simulink model "ofdm_txrx_supermimo"  (Simulink 7.1)
settings: Solver=VariableStepDiscrete  SolverMode=SingleTasking  StartTime=0.0  StopTime=simOnly_simLength  RelTol=1e-3
PostLoadFcn:
  | ofdm_rx_supermimo_init
InitFcn:
  | ofdm_rx_supermimo_init
StartFcn:
  | ofdm_rx_supermimo_init
values from neighbouring files (symbols this model uses):
  Action0_Config = 0* 31*2^18 + 0*2^24 + PHY_AUTORESPONSE_REQ_GOODHDR + PHY_AUTORESPONSE_REQ_GOODPKT + PHY_AUTORESPONSE_REQ_FLAGA    [ofdm_tx_supermimo_init.m]
  Action1_Config = 0* 62*2^18 + PHY_AUTORESPONSE_REQ_GOODHDR + PHY_AUTORESPONSE_REQ_GOODPKT    [ofdm_tx_supermimo_init.m]
  Action2_Config = 0    [ofdm_tx_supermimo_init.m]
  Action3_Config = 0    [ofdm_tx_supermimo_init.m]
  Action4_Config = 0    [ofdm_tx_supermimo_init.m]
  Action5_Config = 0    [ofdm_tx_supermimo_init.m]
  ActionID_AFTransmit = 31    [ofdm_tx_supermimo_init.m]
  ActionID_Disabled = 0    [ofdm_tx_supermimo_init.m]
  ActionID_SetFlagA = 62    [ofdm_tx_supermimo_init.m]
  ActionID_SetFlagB = 61    [ofdm_tx_supermimo_init.m]
  BER_RAM_init_size = 2048    [ofdm_tx_supermimo_init.m]
  CPLength = 16    [ofdm_tx_supermimo_init.m]
  longCorr_coef_i = [-4*real(LTS) 0]    [ofdm_rx_supermimo_init.m]
  longCorr_coef_q = [4*imag(LTS) 0]    [ofdm_rx_supermimo_init.m]
  Match0_Config = 0    [ofdm_tx_supermimo_init.m]
  Match1_Config = 0    [ofdm_tx_supermimo_init.m]
  Match2_Config = 0    [ofdm_tx_supermimo_init.m]
  Match3_Config = 0    [ofdm_tx_supermimo_init.m]
  Match4_Config = 0    [ofdm_tx_supermimo_init.m]
  Match5_Config = 0    [ofdm_tx_supermimo_init.m]
  Match6_Config = 0    [ofdm_tx_supermimo_init.m]
  Match7_Config = 0    [ofdm_tx_supermimo_init.m]
  max_CP_length = 16    [ofdm_tx_supermimo_init.m]
  max_num_baseRateSymbols = 31    [ofdm_tx_supermimo_init.m]
  max_num_subcarriers = 64    [ofdm_tx_supermimo_init.m]
  max_num_trainingSymbols = 15    [ofdm_tx_supermimo_init.m]
  max_numBytes = 16384    [ofdm_tx_supermimo_init.m]
  max_OFDM_symbols = 511    [ofdm_tx_supermimo_init.m]
  modConstellation_qam16 = .75*[1 3 -1 -3]./3    [ofdm_tx_supermimo_init.m]
  modConstellation_qam64 = 0.875*[3 1 5 7 -3 -1 -5 -7]./7    [ofdm_tx_supermimo_init.m]
  modConstellation_qpsk = [1 -1]./sqrt(2)    [ofdm_tx_supermimo_init.m]
  numBaseRateSymbols = ceil(numHeaderBytes / numBytes_BaseRateOFDMSymbol)    [ofdm_tx_supermimo_init.m]
  numSubcarriers = 64    [ofdm_tx_supermimo_init.m]
  numTrainingSymbols = 2    [ofdm_tx_supermimo_init.m]
  pilot_indicies = 7 + ( (64-21) * 2^8) + ( (64-7) * 2^16) + (21 * 2^24)    (= 356068103)    [ofdm_tx_supermimo_init.m]
  pilotValues = hex2dec('A57D') + (2^16 * hex2dec('5A82'))    [ofdm_tx_supermimo_init.m]
  pkt_crossCorr_thresh = 0.7    [ofdm_rx_supermimo_init.m]
  pkt_energy_thresh = 0    [ofdm_rx_supermimo_init.m]
  pktByteNums = numHeaderBytes +  				byteIndex_numPayloadBytes(1)*2^8 +  				byteIndex_numPayloadBytes(2)*2^16 +  				byteIndex_simpleDynModMasks * 2^24    [ofdm_rx_supermimo_init.m]
  PktDet_Delay = 58+32 +      Symbol_Timing_Offset*2^7 +      0*2^16 +      (117* 2^24)    (= 1962935642)    [ofdm_rx_supermimo_init.m]
  pktTiming_count_payloadStart = pktTiming_count_lsStart + pktTiming_ls_num*pktTiming_ls_length    [ofdm_rx_supermimo_init.m]
  preamble = preamble_scale*[shortsyms_10 longsyms_2]    [ofdm_tx_supermimo_init.m]
  preamble_ROM = preamble_I_ROM + preamble_Q_ROM*2^16    [ofdm_tx_supermimo_init.m]
  RAM_init_size = max_numBytes    (= 16384)    [ofdm_tx_supermimo_init.m]
  reg_AF_Tx_Blanking = (320+80) + ( (320+80+79)*2^16)    (= 31392144)    [ofdm_rx_supermimo_init.m]
  reg_AF_Tx_Scaling = 2944    [ofdm_rx_supermimo_init.m]
  reg_Interrupt_PktBuf_Control = reg_InterruptControl +  	1 * 2^16 +  	0 * 2^24    (= 65656)    [ofdm_rx_supermimo_init.m]
  reg_PilotCalcParams = (4 * 2^16) + (minPilotChanMag)    (= 262184.96)    [ofdm_rx_supermimo_init.m]
  regRx_chanEst_minMag = ((0.5)*2^15) + ((0.5 * 2^15)*2^16)    (= 1073758208)    [ofdm_rx_supermimo_init.m]
  regRx_coarseCalcCorrection = round((1.3e-3 - 1.2982e-3)*2^32)    [ofdm_rx_supermimo_init.m]
  regRx_pilotCalcCorrection = round(1.00115 * 2^31)    [ofdm_rx_supermimo_init.m]
  regRx_preCFOoptions = 0 * 2^0 +  	1 * 2^1 +  	0    (= 2)    [ofdm_rx_supermimo_init.m]
  rx_controlBits = 1 * 2  		 + 1 * 4  		 + 0 * 8  		 + 1 * 16 * rx_SISO_Mode  		 + 1 * 32  		 + 1 * 64  		 + 1 * 128  		 + 1 * 256  		 + 1 * 512  		 + 1 * 1024  		 + 1 * 4096  		 + 0 * 16384  		 + 0 * 32768  		 + 1 * 65536  		 + 0 * 131072  		 + 0 * 262144  		 + 1 * 524288  		 + 1 * 1048576 * tx_Alamouti_Mode  		 + 0 * 2097152           + 1 * 33554432  		 + 0 * 4194304  		 + 0 * 8388608  		 + 0 * 16777216  		 + 1 *  ...(+49 chars)    [ofdm_rx_supermimo_init.m]
  Rx_PktDet_LongCorr_Params = LongCorrCounterLoad + 0*2^8 + 0*2^16 + 255*2^24    (= 4278190325)    [ofdm_rx_supermimo_init.m]
  Rx_PktDet_LongCorrThresholds = 8000    [ofdm_rx_supermimo_init.m]
  rx_postEq_scaling = round(rxScaling*2^11) * (1 + 2^16)    [ofdm_rx_supermimo_init.m]
  RxDataScrambling_Seq = [40 198 78 63 82 173 102 245 48 111 172 115 147 230 216 93 72 65 62 2 205 242 122 90 128 83 105 97 73 10 5 252]    [ofdm_tx_supermimo_init.m]
  RxReg_FixedPktLen = 0    [ofdm_rx_supermimo_init.m]
  shortSymbol_time = shortSymbol_time(1:16).'    [ofdm_tx_supermimo_init.m]
  simOnly_numSamples = length(preamble)+( (numSubcarriers+CPLength)*(numTrainingSymbols + numBaseRateSymbols + numFullRateSymbols/2) )    [ofdm_tx_supermimo_init.m]
  train = [train train]    [ofdm_tx_supermimo_init.m]
  tx_controlBits = (antB_preambleShift * 2^4) + (2^1 * tx_Alamouti_Mode) +  	(2^0 * tx_SISO_Mode) + 4*0 + 8*1 + 0*256 + 512*0 + 1024*0 +  	0*hex2dec('1000') + 0*hex2dec('2000') + 0*hex2dec('4000') +  	1*hex2dec('8000') + 1*hex2dec('10000') + hex2dec('20000') +  	16*hex2dec('100000')    [ofdm_tx_supermimo_init.m]
  tx_delays = (50*2^0) + (0*2^8) + (30*2^12)    (= 122930)    [ofdm_tx_supermimo_init.m]
  tx_scaling = (3072) + (12288 * 2^16)    (= 805309440)    [ofdm_tx_supermimo_init.m]
  TxDataScrambling_Seq = [40 198 78 63 82 173 102 245 48 111 172 115 147 230 216 93 72 65 62 2 205 242 122 90 128 83 105 97 73 10 5 252]    [ofdm_tx_supermimo_init.m]
  txfilt_firpm = 0.95*txfilt_firpm./(max(txfilt_firpm))    [ofdm_tx_supermimo_init.m]
  txfilt_intfilt = [0 intfilt(4,4,.8)]    [ofdm_tx_supermimo_init.m]
  txReg_symbolCounts = (2^8 *numBaseRateSymbols ) + numTrainingSymbols    [ofdm_tx_supermimo_init.m]
  TxRx_FFTScaling = (3 * 2^0) +  	(2 * 2^2) +  	(1 * 2^4) +  	(1 * 2^6) +  	(1 * 2^8) +  	(0 * 2^10)    (= 347)    [ofdm_tx_supermimo_init.m]
undefined symbols (not defined in the file or in its folder): AntA, AntB, BaseRate, Down, gcbh, numBuffs, numBytes, Up, xlBlockLoadCallback, xlGetSimulinkPeriod, xlipgetpartsetting
libraries: xbsIndex_r4 x6035, simulink x4, dspsigops x4, commchan3 x2, dspsrcs4 x2, commrflib2 x2
(part 20 of 61)

== system ofdm_txrx_supermimo/OFDM Rx MIMO/FFT & Chan Est/Addr Gen1/Oscillator ==
  en : Inport  OutDataType=sfix(16)  OutScaling=2^0
  rst : Inport  Port=2  OutDataType=sfix(16)  OutScaling=2^0
  Assert : xbsIndex_r4/Assert  Orientation=left  assert_type=on  type_source=Explicitly  arith_type=Boolean  n_bits=16  bin_pt=14  assert_rate=on  rate_source=Explicitly  period=2  output_port=on  block_type=assert
      settings: infoedit=Asserts a user-defined sample rate and/or type on a signal.<P><P>Hardware notes: In hardware this block costs nothing.  block_version=VER_STRING_GOES_HERE
  Inverter1 : xbsIndex_r4/Inverter  Orientation=left  en=off  latency=0  dbl_ovrd=off  block_type=inv
      settings: block_version=VER_STRING_GOES_HERE
  Register : xbsIndex_r4/Register  init=0  rst=on  en=on  dbl_ovrd=off  block_type=register
      settings: block_version=VER_STRING_GOES_HERE
  Q : Outport  OutDataType=sfix(16)  OutScaling=2^0
  wires:
  Register/1 -> Q/1, Assert/1
  en/1 -> Register/3
  Inverter1/1 -> Register/1
  rst/1 -> Register/2
  Assert/1 -> Inverter1/1

== system ofdm_txrx_supermimo/OFDM Rx MIMO/FFT & Chan Est/Addr Gen1/Output Sym Counter ==
  FFT Done : Inport  OutDataType=sfix(16)  OutScaling=2^0
  Not Training : Inport  Port=2  OutDataType=sfix(16)  OutScaling=2^0
  Payload : Inport  Port=3  OutDataType=sfix(16)  OutScaling=2^0
  Read Addr : Inport  Port=4  OutDataType=sfix(16)  OutScaling=2^0
  Constant1 : xbsIndex_r4/Constant  arith_type=Unsigned  const=numSubcarriers-1  n_bits=ceil(log2(numSubcarriers))  bin_pt=0  explicit_period=off  period=1  equ=P=C  opselect=C  inp2=PCIN>>17  opr=+  inp1=P  carry=CIN  dbl_ovrd=off  block_type=constant
      settings: block_version=VER_STRING_GOES_HERE
  Counter1 : xbsIndex_r4/Counter  cnt_type=Free Running  cnt_to=127  operation=Up  start_count=0  cnt_by_val=1  arith_type=Unsigned  n_bits=ceil(log2(max_OFDM_symbols))  bin_pt=0  load_pin=off  rst=on  en=on  explicit_period=off  period=2  dbl_ovrd=off  use_behavioral_HDL=off  use_rpm=on  implementation=Fabric  block_type=counter
      settings: xl_area=[6 12 0 12 0 0 0]  block_version=VER_STRING_GOES_HERE
  Inverter3 : xbsIndex_r4/Inverter  en=off  latency=0  dbl_ovrd=off  block_type=inv
      settings: block_version=VER_STRING_GOES_HERE
  Logical1 : xbsIndex_r4/Logical  logical_function=AND  inputs=2  en=off  latency=0  precision=Full  arith_type=Unsigned  n_bits=8  bin_pt=2  align_bp=on  dbl_ovrd=off  block_type=logical
      settings: xl_area=[0, 0, 0, 0, 0, 0, 0]  block_version=VER_STRING_GOES_HERE
  Logical2 : xbsIndex_r4/Logical  logical_function=OR  inputs=2  en=off  latency=0  precision=Full  arith_type=Unsigned  n_bits=8  bin_pt=2  align_bp=on  dbl_ovrd=off  block_type=logical
      settings: xl_area=[0, 0, 0, 0, 0, 0, 0]  block_version=VER_STRING_GOES_HERE
  Logical3 : xbsIndex_r4/Logical  logical_function=AND  inputs=2  en=off  latency=0  precision=Full  arith_type=Unsigned  n_bits=8  bin_pt=2  align_bp=on  dbl_ovrd=off  block_type=logical
      settings: xl_area=[0, 0, 0, 0, 0, 0, 0]  block_version=VER_STRING_GOES_HERE
  Logical4 : xbsIndex_r4/Logical  logical_function=AND  inputs=2  en=off  latency=0  precision=Full  arith_type=Unsigned  n_bits=8  bin_pt=2  align_bp=on  dbl_ovrd=off  block_type=logical
      settings: xl_area=[0, 0, 0, 0, 0, 0, 0]  block_version=VER_STRING_GOES_HERE
  Posedge : Subsystem
  Posedge1 : Subsystem
  Posedge2 : Subsystem
  Posedge3 : Subsystem
  Relational : xbsIndex_r4/Relational  mode=a=b  en=off  latency=0  dbl_ovrd=off  block_type=relational
      settings: block_version=VER_STRING_GOES_HERE
  S-R Latch2 : Subsystem
  Sym Index : Outport  OutDataType=sfix(16)  OutScaling=2^0
  wires:
  Logical4/1 -> Logical3/2
  S-R Latch2/1(D) -> Logical4/2
  Posedge/1(Q) -> S-R Latch2/1(S), Logical4/1
  FFT Done/1 -> Posedge/1(D)
  Counter1/1 -> Sym Index/1
  Not Training/1 -> Inverter3/1, Logical1/2
  Inverter3/1 -> Logical3/1
  Payload/1 -> Posedge1/1(D)
  Posedge1/1(Q) -> S-R Latch2/2(R), Counter1/1
  Read Addr/1 -> Relational/2
  Constant1/1 -> Relational/1
  Relational/1 -> Logical1/1
  Logical1/1 -> Posedge2/1(D)
  Logical3/1 -> Posedge3/1(D)
  Logical2/1 -> Counter1/2
  Posedge2/1(Q) -> Logical2/1
  Posedge3/1(Q) -> Logical2/2

== system ofdm_txrx_supermimo/OFDM Rx MIMO/FFT & Chan Est/Addr Gen1/Rd Delay ==
  note: Delay the equalizer inputs in Alamout mode in order
      | to calculate pilot phaes for both r0 and r1 before
      | starting the decoding process. In SISO/Multiplexing mode,
      | each symbol has its own pilots, so there's a shorter delay.
  D : Inport
  Delay1 : xbsIndex_r4/Delay  en=off  latency=3  dbl_ovrd=off  reg_retiming=off  block_type=delay
      settings: block_version=10.1.2
  Delay2 : xbsIndex_r4/Delay  en=off  latency=35  dbl_ovrd=off  reg_retiming=off  block_type=delay
      settings: block_version=10.1.2
  Inverter1 : xbsIndex_r4/Inverter  en=off  latency=0  dbl_ovrd=off  block_type=inv
  Logical1 : xbsIndex_r4/Logical  logical_function=AND  inputs=2  en=off  latency=0  precision=Full  arith_type=Unsigned  n_bits=16  bin_pt=0  align_bp=on  dbl_ovrd=off  block_type=logical
  Logical2 : xbsIndex_r4/Logical  logical_function=OR  inputs=2  en=off  latency=0  precision=Full  arith_type=Unsigned  n_bits=16  bin_pt=0  align_bp=on  dbl_ovrd=off  block_type=logical
  Logical3 : xbsIndex_r4/Logical  logical_function=AND  inputs=2  en=off  latency=0  precision=Full  arith_type=Unsigned  n_bits=16  bin_pt=0  align_bp=on  dbl_ovrd=off  block_type=logical
  Q : Outport
  wires:
  Logical1/1 -> Logical2/1
  Logical3/1 -> Logical2/2
  Logical2/1 -> Q/1
  [regRx_AlamoutiMode] -> Logical1/2, Inverter1/1
  Inverter1/1 -> Logical3/1
  Delay2/1 -> Logical1/1
  D/1 -> Delay2/1, Delay1/1
  Delay1/1 -> Logical3/2

== system ofdm_txrx_supermimo/OFDM Rx MIMO/FFT & Chan Est/Addr Gen1/S-R Latch1 ==
  S : Inport  OutDataType=sfix(16)  OutScaling=2^0
  R : Inport  Port=2  OutDataType=sfix(16)  OutScaling=2^0
  Constant2 : xbsIndex_r4/Constant  arith_type=Boolean  const=1  n_bits=1  bin_pt=0  explicit_period=off  period=1  equ=P=C  opselect=C  inp2=PCIN>>17  opr=+  inp1=P  carry=CIN  dbl_ovrd=off  block_type=constant
      settings: block_version=VER_STRING_GOES_HERE
  Register : xbsIndex_r4/Register  init=0  rst=on  en=on  dbl_ovrd=off  block_type=register
      settings: xl_area=[0, 0, 0, 0, 0, 0, 0]  block_version=VER_STRING_GOES_HERE
  D : Outport  OutDataType=sfix(16)  OutScaling=2^0
  wires:
  Register/1 -> D/1
  S/1 -> Register/3
  R/1 -> Register/2
  Constant2/1 -> Register/1

== system ofdm_txrx_supermimo/OFDM Rx MIMO/FFT & Chan Est/Addr_Generator/Initial Read Addr Timing Calc ==
  note: The FFT runs 4x as fast as the input samples. As the input
      | samples are written to the buffers, the FFT waits to start
      | until it can have access to the newest sample it needs
      | 1 cycle after it's written.
  AddSub1 : xbsIndex_r4/AddSub  mode=Addition  use_carryin=off  use_carryout=off  en=off  latency=0  precision=User Defined  arith_type=Unsigned  n_bits=ceil(log2(max_num_subcarriers))+1  bin_pt=0  quantization=Truncate  overflow=Wrap  dbl_ovrd=off  use_behavioral_HDL=on  pipelined=off  use_rpm=on  hw_selection=Fabric  block_type=addsub
      settings: xl_area=[4 0 0 6 0 0 0]  block_version=VER_STRING_GOES_HERE
  Constant1 : xbsIndex_r4/Constant  arith_type=Unsigned  const=0.75*numSubcarriers  n_bits=log2(numSubcarriers)  bin_pt=0  explicit_period=off  period=1  equ=P=C  opselect=C  inp2=PCIN>>17  opr=+  inp1=P  carry=CIN  dbl_ovrd=off  block_type=constant
      settings: block_version=VER_STRING_GOES_HERE
  Up Sample4 : xbsIndex_r4/Up Sample  sample_ratio=2  copy_samples=on  dbl_ovrd=off  block_type=usamp
      settings: infoedit="Up samples input data.  Inserted values  can be zeros or copies of the most recent input sample.<P><P>Hardware notes: No hardware is needed if inserted values are copies of the input sample; otherwise, a mux and single bit flip-flop are used."  block_version=VER_STRING_GOES_HERE
  D : Outport  OutDataType=sfix(16)  OutScaling=2^0
  wires:
  AddSub1/1 -> D/1
  [regRx_symbolTimingOffset] -> Up Sample4/1
  Up Sample4/1 -> AddSub1/2
  Constant1/1 -> AddSub1/1

== system ofdm_txrx_supermimo/OFDM Rx MIMO/FFT & Chan Est/Addr_Generator/Oscillator ==
  en : Inport  OutDataType=sfix(16)  OutScaling=2^0
  rst : Inport  Port=2  OutDataType=sfix(16)  OutScaling=2^0
  Assert : xbsIndex_r4/Assert  Orientation=left  assert_type=on  type_source=Explicitly  arith_type=Boolean  n_bits=16  bin_pt=14  assert_rate=on  rate_source=Explicitly  period=4  output_port=on  block_type=assert
      settings: infoedit=Asserts a user-defined sample rate and/or type on a signal.<P><P>Hardware notes: In hardware this block costs nothing.  block_version=VER_STRING_GOES_HERE
  Inverter1 : xbsIndex_r4/Inverter  Orientation=left  en=off  latency=0  dbl_ovrd=off  block_type=inv
      settings: block_version=VER_STRING_GOES_HERE
  Register1 : xbsIndex_r4/Register  init=0  rst=on  en=on  dbl_ovrd=off  block_type=register
      settings: block_version=VER_STRING_GOES_HERE
  Q : Outport  OutDataType=sfix(16)  OutScaling=2^0
  wires:
  rst/1 -> Register1/2
  Assert/1 -> Inverter1/1
  Inverter1/1 -> Register1/1
  en/1 -> Register1/3
  Register1/1 -> Q/1, Assert/1

== system ofdm_txrx_supermimo/OFDM Rx MIMO/FFT & Chan Est/Addr_Generator/Posedge ==
  D : Inport  OutDataType=sfix(16)  OutScaling=2^0
  Delay : xbsIndex_r4/Delay  en=off  latency=1  dbl_ovrd=off  reg_retiming=off  block_type=delay
      settings: infoedit=Hardware notes: A delay line is a chain, each link of which is an SRL16 followed by a flip-flop. If register retiming is enabled, the delay line is a chain of flip-flops.  xl_area=[1 1 0 0 0 0 0]  block_version=VER_STRING_GOES_HERE
  Inverter : xbsIndex_r4/Inverter  en=off  latency=0  dbl_ovrd=off  block_type=inv
      settings: xl_area=[0 0 0 0 0 0 0]  block_version=VER_STRING_GOES_HERE
  Logical : xbsIndex_r4/Logical  logical_function=AND  inputs=2  en=off  latency=0  precision=Full  arith_type=Unsigned  n_bits=8  bin_pt=2  align_bp=on  dbl_ovrd=off  block_type=logical
      settings: xl_area=[1 0 0 1 0 0 0]  block_version=VER_STRING_GOES_HERE
  Q : Outport  OutDataType=sfix(16)  OutScaling=2^0
  wires:
  Logical/1 -> Q/1
  D/1 -> Delay/1, Logical/1
  Inverter/1 -> Logical/2
  Delay/1 -> Inverter/1

== system ofdm_txrx_supermimo/OFDM Rx MIMO/FFT & Chan Est/Addr_Generator/negedge ==
  D : Inport  OutDataType=sfix(16)  OutScaling=2^0
  Delay : xbsIndex_r4/Delay  en=off  latency=1  dbl_ovrd=off  reg_retiming=off  block_type=delay
      settings: infoedit=Hardware notes: A delay line is a chain, each link of which is an SRL16 followed by a flip-flop. If register retiming is enabled, the delay line is a chain of flip-flops.  xl_area=[1 1 0 0 0 0 0]  block_version=VER_STRING_GOES_HERE
  Inverter : xbsIndex_r4/Inverter  en=off  latency=0  dbl_ovrd=off  block_type=inv
      settings: xl_area=[0 0 0 0 0 0 0]  block_version=VER_STRING_GOES_HERE
  Logical : xbsIndex_r4/Logical  logical_function=AND  inputs=2  en=off  latency=0  precision=Full  arith_type=Unsigned  n_bits=8  bin_pt=2  align_bp=on  dbl_ovrd=off  block_type=logical
      settings: xl_area=[1 0 0 1 0 0 0]  block_version=VER_STRING_GOES_HERE
  Q : Outport  OutDataType=sfix(16)  OutScaling=2^0
  wires:
  Inverter/1 -> Logical/2
  D/1 -> Delay/1, Inverter/1
  Logical/1 -> Q/1
  Delay/1 -> Logical/1

== system ofdm_txrx_supermimo/OFDM Rx MIMO/FFT & Chan Est/Addr_Generator/negedge1 ==
  D : Inport  OutDataType=sfix(16)  OutScaling=2^0
  Delay : xbsIndex_r4/Delay  en=off  latency=1  dbl_ovrd=off  reg_retiming=off  block_type=delay
      settings: infoedit=Hardware notes: A delay line is a chain, each link of which is an SRL16 followed by a flip-flop. If register retiming is enabled, the delay line is a chain of flip-flops.  xl_area=[1 1 0 0 0 0 0]  block_version=VER_STRING_GOES_HERE
  Inverter : xbsIndex_r4/Inverter  en=off  latency=0  dbl_ovrd=off  block_type=inv
      settings: xl_area=[0 0 0 0 0 0 0]  block_version=VER_STRING_GOES_HERE
  Logical : xbsIndex_r4/Logical  logical_function=AND  inputs=2  en=off  latency=0  precision=Full  arith_type=Unsigned  n_bits=8  bin_pt=2  align_bp=on  dbl_ovrd=off  block_type=logical
      settings: xl_area=[1 0 0 1 0 0 0]  block_version=VER_STRING_GOES_HERE
  Q : Outport  OutDataType=sfix(16)  OutScaling=2^0
  wires:
  Inverter/1 -> Logical/1
  Delay/1 -> Logical/2
  D/1 -> Inverter/1, Delay/1
  Logical/1 -> Q/1

== system ofdm_txrx_supermimo/OFDM Rx MIMO/FFT & Chan Est/Channel Estimation & Pilot Processing/Actual Trainig Window ==
  H Vin : Inport
  Train Sym : Inport  Port=2
  Logical2 : xbsIndex_r4/Logical  logical_function=AND  inputs=2  en=off  latency=0  precision=Full  arith_type=Unsigned  n_bits=8  bin_pt=2  align_bp=on  dbl_ovrd=off  block_type=logical
      settings: xl_area=[1 0 0 1 0 0 0]  block_version=VER_STRING_GOES_HERE
  Posedge : Subsystem
  S-R Latch : Subsystem
  negedge : Subsystem
  Train : Outport
  wires:
  S-R Latch/1(D) -> Logical2/1
  Posedge/1(Q) -> S-R Latch/1(S)
  negedge/1(Q) -> S-R Latch/2(R)
  H Vin/1 -> Posedge/1(D)
  Logical2/1 -> Train/1
  Train Sym/1 -> negedge/1(D), Logical2/2

== system ofdm_txrx_supermimo/OFDM Rx MIMO/FFT & Chan Est/Channel Estimation & Pilot Processing/Bus Creator ==
  AA-I : Inport  OutDataType=sfix(16)  OutScaling=2^0
  AA-Q : Inport  Port=2  OutDataType=sfix(16)  OutScaling=2^0
  AB-I : Inport  Port=3  OutDataType=sfix(16)  OutScaling=2^0
  AB-Q : Inport  Port=4  OutDataType=sfix(16)  OutScaling=2^0
  BA-I : Inport  Port=5  OutDataType=sfix(16)  OutScaling=2^0
  BA-Q : Inport  Port=6  OutDataType=sfix(16)  OutScaling=2^0
  BB-I : Inport  Port=7  OutDataType=sfix(16)  OutScaling=2^0
  BB-Q : Inport  Port=8  OutDataType=sfix(16)  OutScaling=2^0
  Bus Creator1 : BusCreator
  Bus Creator2 : BusCreator
  Constant1 : xbsIndex_r4/Constant  arith_type=Signed (2's comp)  const=0  n_bits=18  bin_pt=16  explicit_period=on  period=2  equ=P=C  opselect=C  inp2=PCIN>>17  opr=+  inp1=P  carry=CIN  dbl_ovrd=off  block_type=constant
      settings: block_version=VER_STRING_GOES_HERE
  Constant2 : xbsIndex_r4/Constant  arith_type=Signed (2's comp)  const=0  n_bits=18  bin_pt=16  explicit_period=on  period=2  equ=P=C  opselect=C  inp2=PCIN>>17  opr=+  inp1=P  carry=CIN  dbl_ovrd=off  block_type=constant
      settings: block_version=VER_STRING_GOES_HERE
  I : Outport  OutDataType=sfix(16)  OutScaling=2^0
  Q : Outport  Port=2  OutDataType=sfix(16)  OutScaling=2^0
  wires:
  AA-I/1 -> Bus Creator1/1
  AB-I/1 -> Bus Creator1/2
  Bus Creator1/1 -> I/1
  BA-I/1 -> Bus Creator1/3
  BB-I/1 -> Bus Creator1/4
  AA-Q/1 -> Bus Creator2/1
  AB-Q/1 -> Bus Creator2/2
  Bus Creator2/1 -> Q/1
  BA-Q/1 -> Bus Creator2/3
  BB-Q/1 -> Bus Creator2/4

== system ofdm_txrx_supermimo/OFDM Rx MIMO/FFT & Chan Est/Channel Estimation & Pilot Processing/Chan Est Buffer & Mag Calc ==
  note: Stores each channel coefficient as a
      | 32-bit word, combining [I Q] per word.
  AA-I : Inport  OutDataType=sfix(16)  OutScaling=2^0
  AA-Q : Inport  Port=2  OutDataType=sfix(16)  OutScaling=2^0
  AB-I : Inport  Port=3  OutDataType=sfix(16)  OutScaling=2^0
  AB-Q : Inport  Port=4  OutDataType=sfix(16)  OutScaling=2^0
  BA-I : Inport  Port=5  OutDataType=sfix(16)  OutScaling=2^0
  BA-Q : Inport  Port=6  OutDataType=sfix(16)  OutScaling=2^0
  BB-I : Inport  Port=7  OutDataType=sfix(16)  OutScaling=2^0
  BB-Q : Inport  Port=8  OutDataType=sfix(16)  OutScaling=2^0
  Est Vin : Inport  Port=9  OutDataType=sfix(16)  OutScaling=2^0
  SubCar Index : Inport  Port=10  OutDataType=sfix(16)  OutScaling=2^0
  1MSB : xbsIndex_r4/Slice  nbits=1  boolean_output=on  mode=Upper Bit Location + Width  bit1=0  base1=MSB of Input  bit0=0  base0=LSB of Input  dbl_ovrd=off  block_type=slice
      settings: infoedit="Extracts a given range of bits from each input sample and presents it at the output.  The output type is ordinarily unsigned with binary point at zero, but can be Boolean when the slice is one bit wide.<P><P>Hardware notes: In hardware this block costs nothing."  block_version=VER_STRING_GOES_HERE
  "2x16b -> 32b" : Subsystem
  Concat : xbsIndex_r4/Concat  num_inputs=2  dbl_ovrd=off  block_type=concat
      settings: block_version=10.1.3
  Convert{3..10} (8 alike) : xbsIndex_r4/Convert  arith_type="Signed  (2's comp)"  n_bits=16  bin_pt=14  quantization=Truncate  overflow=Flag as error  latency=0  dbl_ovrd=off  pipeline=off  block_type=convert
      settings: xl_area=[0, 0, 0, 0, 0, 0, 0]  block_version=VER_STRING_GOES_HERE
  Counter : xbsIndex_r4/Counter  cnt_type=Free Running  cnt_to=Inf  operation=Up  start_count=0  cnt_by_val=1  arith_type=Unsigned  n_bits=2  bin_pt=0  load_pin=off  rst=on  en=on  explicit_period=off  period=1  dbl_ovrd=off  use_behavioral_HDL=off  use_rpm=on  implementation=Fabric  block_type=counter
      settings: xl_area=[6 12 0 12 0 0 0]  block_version=VER_STRING_GOES_HERE
  Delay{1..5} (5 alike) : xbsIndex_r4/Delay  en=off  latency=1  dbl_ovrd=off  reg_retiming=off  block_type=delay
      settings: infoedit=Hardware notes: A delay line is a chain, each link of which is an SRL16 followed by a flip-flop. If register retiming is enabled, the delay line is a chain of flip-flops.  xl_area=[0, 0, 0, 0, 0, 0, 0]  block_version=VER_STRING_GOES_HERE
  Logical1 : xbsIndex_r4/Logical  logical_function=AND  inputs=2  en=off  latency=0  precision=Full  arith_type=Unsigned  n_bits=8  bin_pt=2  align_bp=on  dbl_ovrd=off  block_type=logical
      settings: xl_area=[1 0 0 1 0 0 0]  block_version=VER_STRING_GOES_HERE
  Logical2 : xbsIndex_r4/Logical  logical_function=AND  inputs=2  en=off  latency=0  precision=Full  arith_type=Unsigned  n_bits=8  bin_pt=2  align_bp=on  dbl_ovrd=off  block_type=logical
      settings: xl_area=[1 0 0 1 0 0 0]  block_version=VER_STRING_GOES_HERE
  Mux1 : xbsIndex_r4/Mux  inputs=4  en=off  latency=1  precision=Full  arith_type="Signed  (2's comp)"  n_bits=8  bin_pt=2  quantization=Truncate  overflow=Wrap  dbl_ovrd=off  block_type=mux
      settings: xl_area=[0, 0, 0, 0, 0, 0, 0]  block_version=VER_STRING_GOES_HERE
  Mux2 : xbsIndex_r4/Mux  inputs=4  en=off  latency=1  precision=Full  arith_type="Signed  (2's comp)"  n_bits=8  bin_pt=2  quantization=Truncate  overflow=Wrap  dbl_ovrd=off  block_type=mux
      settings: xl_area=[0, 0, 0, 0, 0, 0, 0]  block_version=VER_STRING_GOES_HERE
  Negedge : Subsystem
  Scope : Scope  NumInputPorts=6
      settings: YMin=-5~-5~-5~-5~-5~-5  YMax=5~5~5~5~5~5  SaveName=ScopeData6
  Shared Memory : xbsIndex_r4/Shared Memory  shared_memory_name='ChannelEstimates'  depth=numSubcarriers * 4  ownership=Locally Owned and Initialized  initVector=zeros(1,numSubcarriers*4)  mutex=Unprotected  mode=Read and Write  write_mode=Read After Write  time_out=0  latency=1  explicit_data_type=on  arith_type=Unsigned  n_bits=32  bin_pt=0  implementation=Block RAM  use_rpm=off  block_type=shmem
      settings: AttributesFormatString=<< %<shared_memory_name> >>  block_version=10.1.3
  done{1..5,9} (6 alike) : xbsIndex_r4/Gateway Out  hdl_port=off  timing_constraint=None  locs_specified=off  LOCs={}  block_type=gatewayout
      settings: infoedit="Gateway out block.  Converts Xilinx fixed point inputs into ouputs of type Simulink integer, double, or fixed point.<P><P>Hardware notes:  In hardware these blocks become top level output ports or are discarded, depending on how they are configured."  xl_area=[0 0 0 0 1 0 0]  block_version=VER_STRING_GOES_HERE
  edge : Subsystem
  wires:
  SubCar Index/1 -> Delay1/1, 1MSB/1, done1/1
  Est Vin/1 -> edge/1(D), Logical1/1, Logical2/2
  1MSB/1 -> Negedge/1(D)
  Negedge/1(Q) -> Logical1/2
  Logical1/1 -> Counter/2
  edge/1(Q) -> Counter/1
  "2x16b -> 32b"/1("[I Q]") -> Shared Memory/2
  Concat/1 -> Delay2/1
  Counter/1 -> Mux2/1, Mux1/1, done5/1, Concat/1
  AA-I/1 -> Convert3/1
  AB-I/1 -> Convert4/1
  BA-I/1 -> Convert5/1
  BB-I/1 -> Convert6/1
  AA-Q/1 -> Convert7/1
  AB-Q/1 -> Convert8/1
  BA-Q/1 -> Convert9/1
  BB-Q/1 -> Convert10/1
  Convert7/1 -> Mux1/2
  Convert8/1 -> Mux1/3
  Convert9/1 -> Mux1/4
  Convert10/1 -> Mux1/5
  Convert3/1 -> Mux2/2
  Convert4/1 -> Mux2/3
  Convert5/1 -> Mux2/4
  Convert6/1 -> Mux2/5
  Mux2/1 -> "2x16b -> 32b"/1(I), done9/1
  Delay1/1 -> Concat/2
  Mux1/1 -> "2x16b -> 32b"/2(Q)
  Delay2/1 -> Delay3/1
  Delay3/1 -> Shared Memory/1, done2/1
  done9/1 -> Scope/1
  done1/1 -> Scope/2
  done2/1 -> Scope/3
  done3/1 -> Scope/4
  done4/1 -> Scope/5
  done5/1 -> Scope/6
  Logical2/1 -> Delay5/1
  [regRx_chanEst_RecordEn] -> Logical2/1
  Delay4/1 -> Shared Memory/3, done4/1
  Delay5/1 -> Delay4/1
  "2x16b -> 32b"/2(H_Mag) -> done3/1

== system ofdm_txrx_supermimo/OFDM Rx MIMO/FFT & Chan Est/Channel Estimation & Pilot Processing/Downsamplng ==
  AA-I : Inport  OutDataType=sfix(16)  OutScaling=2^0
  AA-Q : Inport  Port=2  OutDataType=sfix(16)  OutScaling=2^0
  AB-I : Inport  Port=3  OutDataType=sfix(16)  OutScaling=2^0
  AB-Q : Inport  Port=4  OutDataType=sfix(16)  OutScaling=2^0
  BA-I : Inport  Port=5  OutDataType=sfix(16)  OutScaling=2^0
  BA-Q : Inport  Port=6  OutDataType=sfix(16)  OutScaling=2^0
  BB-I : Inport  Port=7  OutDataType=sfix(16)  OutScaling=2^0
  BB-Q : Inport  Port=8  OutDataType=sfix(16)  OutScaling=2^0
  Down Sample{,1..7} (8 alike) : xbsIndex_r4/Down Sample  sample_ratio=2  sample_phase="Last Value of Frame  (most efficient)"  en=off  latency=1  dbl_ovrd=off  block_type=dsamp
      settings: block_version=10.1.2
  " AA-I" : Outport  OutDataType=sfix(16)  OutScaling=2^0
  " AA-Q" : Outport  Port=2  OutDataType=sfix(16)  OutScaling=2^0
  " AB-I" : Outport  Port=3  OutDataType=sfix(16)  OutScaling=2^0
  " AB-Q" : Outport  Port=4  OutDataType=sfix(16)  OutScaling=2^0
  " BA-I" : Outport  Port=5  OutDataType=sfix(16)  OutScaling=2^0
  " BA-Q" : Outport  Port=6  OutDataType=sfix(16)  OutScaling=2^0
  " BB-I" : Outport  Port=7  OutDataType=sfix(16)  OutScaling=2^0
  " BB-Q" : Outport  Port=8  OutDataType=sfix(16)  OutScaling=2^0
  wires:
  AA-I/1 -> Down Sample/1
  AB-I/1 -> Down Sample1/1
  AA-Q/1 -> Down Sample4/1
  BA-I/1 -> Down Sample2/1
  AB-Q/1 -> Down Sample5/1
  BB-I/1 -> Down Sample3/1
  BA-Q/1 -> Down Sample6/1
  BB-Q/1 -> Down Sample7/1
  Down Sample/1 -> " AA-I"/1
  Down Sample1/1 -> " AB-I"/1
  Down Sample2/1 -> " BA-I"/1
  Down Sample3/1 -> " BB-I"/1
  Down Sample4/1 -> " AA-Q"/1
  Down Sample5/1 -> " AB-Q"/1
  Down Sample6/1 -> " BA-Q"/1
  Down Sample7/1 -> " BB-Q"/1

== system ofdm_txrx_supermimo/OFDM Rx MIMO/FFT & Chan Est/Channel Estimation & Pilot Processing/H_A-A Estimate Buffer ==
  I : Inport  OutDataType=sfix(16)  OutScaling=2^0
  Q : Inport  Port=2  OutDataType=sfix(16)  OutScaling=2^0
  Est_WE : Inport  Port=3  OutDataType=sfix(16)  OutScaling=2^0
  Est_Reset : Inport  Port=4  OutDataType=sfix(16)  OutScaling=2^0
  FFT_Addr : Inport  Port=5  OutDataType=sfix(16)  OutScaling=2^0
  EQ_Addr : Inport  Port=6  OutDataType=sfix(16)  OutScaling=2^0
  I Estimate Buffer : Subsystem
  Q Estimate Buffer : Subsystem
  EstI_fast : Outport  OutDataType=sfix(16)  OutScaling=2^0
  EstQ_fast : Outport  Port=2  OutDataType=sfix(16)  OutScaling=2^0
  EstI : Outport  Port=3  OutDataType=sfix(16)  OutScaling=2^0
  EstQ : Outport  Port=4  OutDataType=sfix(16)  OutScaling=2^0
  wires:
  Est_Reset/1 -> I Estimate Buffer/4(Rst), Q Estimate Buffer/4(Rst)
  Est_WE/1 -> I Estimate Buffer/3(Est_WE), Q Estimate Buffer/3(Est_WE)
  I Estimate Buffer/1(h[n]) -> EstI/1
  FFT_Addr/1 -> I Estimate Buffer/2(Fast_Addr), Q Estimate Buffer/2(Fast_Addr)
  I/1 -> I Estimate Buffer/1(Est_Data)
  I Estimate Buffer/2(h[n] (fast)) -> EstI_fast/1
  EQ_Addr/1 -> I Estimate Buffer/5(Slow_Addr), Q Estimate Buffer/5(Slow_Addr)
  Q/1 -> Q Estimate Buffer/1(Est_Data)
  Q Estimate Buffer/1(h[n]) -> EstQ/1
  Q Estimate Buffer/2(h[n] (fast)) -> EstQ_fast/1

== system ofdm_txrx_supermimo/OFDM Rx MIMO/FFT & Chan Est/Channel Estimation & Pilot Processing/H_A-B Estimate Buffer ==
  I : Inport  OutDataType=sfix(16)  OutScaling=2^0
  Q : Inport  Port=2  OutDataType=sfix(16)  OutScaling=2^0
  Est_WE : Inport  Port=3  OutDataType=sfix(16)  OutScaling=2^0
  Est_Reset : Inport  Port=4  OutDataType=sfix(16)  OutScaling=2^0
  Xk_Index : Inport  Port=5  OutDataType=sfix(16)  OutScaling=2^0
  I Estimate Buffer : Subsystem
  Q Estimate Buffer : Subsystem
  EstI : Outport  OutDataType=sfix(16)  OutScaling=2^0
  EstQ : Outport  Port=2  OutDataType=sfix(16)  OutScaling=2^0
  wires:
  I/1 -> I Estimate Buffer/1(Est_Data)
  Xk_Index/1 -> I Estimate Buffer/2(Est_Addr), Q Estimate Buffer/2(Est_Addr)
  I Estimate Buffer/1(h[n]) -> EstI/1
  Est_WE/1 -> I Estimate Buffer/3(Est_WE), Q Estimate Buffer/3(Est_WE)
  Est_Reset/1 -> I Estimate Buffer/4(Rst), Q Estimate Buffer/4(Rst)
  Q Estimate Buffer/1(h[n]) -> EstQ/1
  Q/1 -> Q Estimate Buffer/1(Est_Data)

== system ofdm_txrx_supermimo/OFDM Rx MIMO/FFT & Chan Est/Channel Estimation & Pilot Processing/H_B-A Estimate Buffer ==
  I : Inport  OutDataType=sfix(16)  OutScaling=2^0
  Q : Inport  Port=2  OutDataType=sfix(16)  OutScaling=2^0
  Est_WE : Inport  Port=3  OutDataType=sfix(16)  OutScaling=2^0
  Est_Reset : Inport  Port=4  OutDataType=sfix(16)  OutScaling=2^0
  FFT_Addr : Inport  Port=5  OutDataType=sfix(16)  OutScaling=2^0
  EQ_Addr : Inport  Port=6  OutDataType=sfix(16)  OutScaling=2^0
  I Estimate Buffer : Subsystem
  Q Estimate Buffer : Subsystem
  EstI_fast : Outport  OutDataType=sfix(16)  OutScaling=2^0
  EstQ_fast : Outport  Port=2  OutDataType=sfix(16)  OutScaling=2^0
  EstI : Outport  Port=3  OutDataType=sfix(16)  OutScaling=2^0
  EstQ : Outport  Port=4  OutDataType=sfix(16)  OutScaling=2^0
  wires:
  Q Estimate Buffer/2(h[n] (fast)) -> EstQ_fast/1
  Q Estimate Buffer/1(h[n]) -> EstQ/1
  Q/1 -> Q Estimate Buffer/1(Est_Data)
  EQ_Addr/1 -> Q Estimate Buffer/5(Slow_Addr), I Estimate Buffer/5(Slow_Addr)
  I Estimate Buffer/2(h[n] (fast)) -> EstI_fast/1
  I/1 -> I Estimate Buffer/1(Est_Data)
  FFT_Addr/1 -> Q Estimate Buffer/2(Fast_Addr), I Estimate Buffer/2(Fast_Addr)
  I Estimate Buffer/1(h[n]) -> EstI/1
  Est_WE/1 -> Q Estimate Buffer/3(Est_WE), I Estimate Buffer/3(Est_WE)
  Est_Reset/1 -> Q Estimate Buffer/4(Rst), I Estimate Buffer/4(Rst)

== system ofdm_txrx_supermimo/OFDM Rx MIMO/FFT & Chan Est/Channel Estimation & Pilot Processing/H_B-B Estimate Buffer ==
  I : Inport  OutDataType=sfix(16)  OutScaling=2^0
  Q : Inport  Port=2  OutDataType=sfix(16)  OutScaling=2^0
  Est_WE : Inport  Port=3  OutDataType=sfix(16)  OutScaling=2^0
  Est_Reset : Inport  Port=4  OutDataType=sfix(16)  OutScaling=2^0
  Xk_Index : Inport  Port=5  OutDataType=sfix(16)  OutScaling=2^0
  I Estimate Buffer : Subsystem
  Q Estimate Buffer : Subsystem
  EstI : Outport  OutDataType=sfix(16)  OutScaling=2^0
  EstQ : Outport  Port=2  OutDataType=sfix(16)  OutScaling=2^0
  wires:
  Q/1 -> Q Estimate Buffer/1(Est_Data)
  Q Estimate Buffer/1(h[n]) -> EstQ/1
  Est_Reset/1 -> Q Estimate Buffer/4(Rst), I Estimate Buffer/4(Rst)
  Est_WE/1 -> Q Estimate Buffer/3(Est_WE), I Estimate Buffer/3(Est_WE)
  I Estimate Buffer/1(h[n]) -> EstI/1
  Xk_Index/1 -> Q Estimate Buffer/2(Est_Addr), I Estimate Buffer/2(Est_Addr)
  I/1 -> I Estimate Buffer/1(Est_Data)

== system ofdm_txrx_supermimo/OFDM Rx MIMO/FFT & Chan Est/Channel Estimation & Pilot Processing/Mult by training ==
  AR : Inport  OutDataType=sfix(16)  OutScaling=2^0
  AI : Inport  Port=2  OutDataType=sfix(16)  OutScaling=2^0
  BR : Inport  Port=3  OutDataType=sfix(16)  OutScaling=2^0
  Constant1 : xbsIndex_r4/Constant  arith_type=Signed (2's comp)  const=0  n_bits=2  bin_pt=1  explicit_period=off  period=1  equ=P=C  opselect=C  inp2=PCIN>>17  opr=+  inp1=P  carry=CIN  dbl_ovrd=off  block_type=constant
      settings: block_version=VER_STRING_GOES_HERE
  Constant2 : xbsIndex_r4/Constant  arith_type=Signed (2's comp)  const=0  n_bits=2  bin_pt=1  explicit_period=off  period=1  equ=P=C  opselect=C  inp2=PCIN>>17  opr=+  inp1=P  carry=CIN  dbl_ovrd=off  block_type=constant
      settings: block_version=VER_STRING_GOES_HERE
  Mux3 : xbsIndex_r4/Mux  inputs=3  en=off  latency=1  precision=Full  arith_type="Signed  (2's comp)"  n_bits=8  bin_pt=2  quantization=Truncate  overflow=Wrap  dbl_ovrd=off  block_type=mux
      settings: xl_area=[0, 0, 0, 0, 0, 0, 0]  block_version=VER_STRING_GOES_HERE
  Mux4 : xbsIndex_r4/Mux  inputs=3  en=off  latency=1  precision=Full  arith_type="Signed  (2's comp)"  n_bits=8  bin_pt=2  quantization=Truncate  overflow=Wrap  dbl_ovrd=off  block_type=mux
      settings: xl_area=[0, 0, 0, 0, 0, 0, 0]  block_version=VER_STRING_GOES_HERE
  Negate : xbsIndex_r4/Negate  precision=User Defined  arith_type="Signed  (2's comp)"  n_bits=16  bin_pt=15  quantization=Truncate  overflow=Flag as error  en=off  latency=0  dbl_ovrd=off  block_type=negate
      settings: xl_area=[0, 0, 0, 0, 0, 0, 0]  block_version=VER_STRING_GOES_HERE
  Negate1 : xbsIndex_r4/Negate  precision=User Defined  arith_type="Signed  (2's comp)"  n_bits=16  bin_pt=15  quantization=Truncate  overflow=Flag as error  en=off  latency=0  dbl_ovrd=off  block_type=negate
      settings: xl_area=[0, 0, 0, 0, 0, 0, 0]  block_version=VER_STRING_GOES_HERE
  Reinterpret : xbsIndex_r4/Reinterpret  force_arith_type=on  arith_type=Unsigned  force_bin_pt=on  bin_pt=0  block_type=cast
      settings: infoedit="Changes type of samples without altering their binary representation.<P><P>Hardware notes: In hardware this block costs nothing.<P><P>Example:  Suppose the input is 6 bits wide, signed, with 2 fractional bits, and the output is forced to unsigned with 0 fractional bits.  Then an input of -2.0 (1110.00 in binary 2's complement) becomes an output of 56 (111000 in binary)."  block_version=VER_STRING_GOES_HERE
  PR : Outport  OutDataType=sfix(16)  OutScaling=2^0
  PI : Outport  Port=2  OutDataType=sfix(16)  OutScaling=2^0
  wires:
  Reinterpret/1 -> Mux4/1, Mux3/1
  Mux3/1 -> PR/1
  Negate/1 -> Mux3/4
  Constant2/1 -> Mux3/2
  Mux4/1 -> PI/1
  Negate1/1 -> Mux4/4
  Constant1/1 -> Mux4/2
  AI/1 -> Negate1/1, Mux4/3
  BR/1 -> Reinterpret/1
  AR/1 -> Mux3/3, Negate/1

== system ofdm_txrx_supermimo/OFDM Rx MIMO/FFT & Chan Est/Channel Estimation & Pilot Processing/Pilot Processing ==
  I : Inport
  Q : Inport  Port=2
  Xk_Index : Inport  Port=3
  Sym_Index : Inport  Port=4
  Train : Inport  Port=5
  HAA_I : Inport  Port=6
  HAA_Q : Inport  Port=7
  HBA_I : Inport  Port=8
  HBA_Q : Inport  Port=9
  CFO Calculation : Subsystem
  Convert3 : xbsIndex_r4/Convert  arith_type="Signed  (2's comp)"  n_bits=14  bin_pt=13  quantization=Truncate  overflow=Wrap  latency=0  dbl_ovrd=off  pipeline=off  block_type=convert
      settings: xl_area=[0, 0, 0, 0, 0, 0, 0]  block_version=VER_STRING_GOES_HERE
  Delay12 : xbsIndex_r4/Delay  en=off  latency=2  dbl_ovrd=off  reg_retiming=off  block_type=delay
      settings: infoedit=Hardware notes: A delay line is a chain, each link of which is an SRL16 followed by a flip-flop. If register retiming is enabled, the delay line is a chain of flip-flops.  xl_area=[0, 0, 0, 0, 0, 0, 0]  block_version=VER_STRING_GOES_HERE
  Delay13 : xbsIndex_r4/Delay  en=off  latency=2  dbl_ovrd=off  reg_retiming=off  block_type=delay
      settings: infoedit=Hardware notes: A delay line is a chain, each link of which is an SRL16 followed by a flip-flop. If register retiming is enabled, the delay line is a chain of flip-flops.  xl_area=[0, 0, 0, 0, 0, 0, 0]  block_version=VER_STRING_GOES_HERE
  Delay14 : xbsIndex_r4/Delay  en=off  latency=1  dbl_ovrd=off  reg_retiming=off  block_type=delay
      settings: infoedit=Hardware notes: A delay line is a chain, each link of which is an SRL16 followed by a flip-flop. If register retiming is enabled, the delay line is a chain of flip-flops.  xl_area=[0, 0, 0, 0, 0, 0, 0]  block_version=VER_STRING_GOES_HERE
  Phase Noise Tracker : Subsystem
  Pilot Phase Error Calc : Subsystem
  Register2 : xbsIndex_r4/Register  init=0  rst=on  en=on  dbl_ovrd=off  block_type=register
      settings: block_version=8.2.02
  Reinterpret : xbsIndex_r4/Reinterpret  force_arith_type=on  arith_type=Unsigned  force_bin_pt=on  bin_pt=0  block_type=reinterpret
      settings: infoedit="Changes signal type without altering the binary representation.   You can changed the signal between signed and unsigned, and relocate the binary point.<P><P>Hardware notes: In hardware this block costs nothing.<P><P>Example:  Suppose the input is 6 bits wide, signed, with 2 fractional bits, and the output is forced to unsigned with 0 fractional bits.  Then an input of -2.0 (1110.00 in binary 2's complement) becomes an output of 56 (111000 in binary)."  block_version=10.1
  Rx_debug_PhaseError : xbsIndex_r4/Gateway Out  hdl_port=on  timing_constraint=None  locs_specified=off  LOCs={}  block_type=gatewayout
      settings: infoedit="Gateway out block.  Converts Xilinx fixed point inputs into ouputs of type Simulink integer, double, or fixed point.<P><P>Hardware notes:  In hardware these blocks become top level output ports or are discarded, depending on how they are configured."  xl_area=[0 0 0 0 1 0 0]  block_version=VER_STRING_GOES_HERE
  Phase I : Outport  OutDataType=sfix(16)  OutScaling=2^0
  Phase Q : Outport  Port=2  OutDataType=sfix(16)  OutScaling=2^0
  tag scope: PilotPhaseErr=global, preSpin_pilotCFOest=global, preSpin_pilotCFOest_valid=global, regRx_pilotCFOest=global, regRx_pilotCFOest_en=global
  wires:
  Pilot Phase Error Calc/2(Rst) -> Register2/2, CFO Calculation/3(Rst), Phase Noise Tracker/2(Reset)
  Delay12/1 -> Pilot Phase Error Calc/1(Raw I)
  Delay13/1 -> Pilot Phase Error Calc/2(Raw Q)
  Delay14/1 -> Pilot Phase Error Calc/4(Sym Index)
  I/1 -> Delay12/1
  Q/1 -> Delay13/1
  Xk_Index/1 -> Pilot Phase Error Calc/3(Xk_index)
  Sym_Index/1 -> Delay14/1
  Train/1 -> Pilot Phase Error Calc/9(Train)
  Register2/1 -> [PilotPhaseErr], Convert3/1
  HAA_I/1 -> Pilot Phase Error Calc/5(H_AA I)
  HAA_Q/1 -> Pilot Phase Error Calc/6(H_AA Q)
  HBA_I/1 -> Pilot Phase Error Calc/7(H_BA I)
  HBA_Q/1 -> Pilot Phase Error Calc/8(H_BA Q)
  Pilot Phase Error Calc/3(Vout) -> Register2/3, CFO Calculation/2(Vin), Phase Noise Tracker/3(Vin)
  Pilot Phase Error Calc/1(Phase Err) -> Register2/1, CFO Calculation/1(Phase Err), Phase Noise Tracker/1(Phase Err)
  Convert3/1 -> Rx_debug_PhaseError/1
  Phase Noise Tracker/1(Phases_I) -> Phase I/1
  Phase Noise Tracker/2(Phases_Q) -> Phase Q/1
  Pilot Phase Error Calc/4(" Sym Index") -> Phase Noise Tracker/4(Sym Index)
  CFO Calculation/1(CFO) -> [preSpin_pilotCFOest], Reinterpret/1
  CFO Calculation/2(Vout) -> [regRx_pilotCFOest_en], [preSpin_pilotCFOest_valid]
  Reinterpret/1 -> [regRx_pilotCFOest]

== system ofdm_txrx_supermimo/OFDM Rx MIMO/FFT & Chan Est/Channel Estimation & Pilot Processing/Training? ==
  note: For SISO/multiplexing modes, numTrainingSymols is
      | doubled, as the FFT is run twice for each over-the-air
      | OFDM symbol period. For Alamouti mode, the FFT is
      | only run once per symbol, so numTrainingSymbols is
      | used as-is.
  note: The channel estimate buffers calculate the
      | running sum of estimates per subcarrier. In the first
      | training interval, the old estimates need to be ingored
      | so that the actual first estimate becomes the starting value
      | for the running sum. In SISO mode, the first training
      | interval lasts 2 FFTs (symIndex=[0 1]). In MIMO
      | mode, it lasts four (symIndex[0 1 2 3]).
  SynIndex : Inport
  Clear2 : xbsIndex_r4/Constant  arith_type=Unsigned  const=1  n_bits=1  bin_pt=0  explicit_period=off  period=1  equ=P=C  opselect=C  inp2=PCIN>>17  opr=+  inp1=P  carry=CIN  dbl_ovrd=off  block_type=constant
      settings: block_version=VER_STRING_GOES_HERE
  Concat : xbsIndex_r4/Concat  num_inputs=2  dbl_ovrd=off  block_type=concat
      settings: block_version=8.2.01
  Inverter1 : xbsIndex_r4/Inverter  en=off  latency=0  dbl_ovrd=off  block_type=inv
      settings: block_version=VER_STRING_GOES_HERE
  Inverter2 : xbsIndex_r4/Inverter  en=off  latency=0  dbl_ovrd=off  block_type=inv
      settings: block_version=VER_STRING_GOES_HERE
  Logical2 : xbsIndex_r4/Logical  logical_function=AND  inputs=2  en=off  latency=0  precision=Full  arith_type=Unsigned  n_bits=8  bin_pt=2  align_bp=on  dbl_ovrd=off  block_type=logical
      settings: xl_area=[1 0 0 1 0 0 0]  block_version=VER_STRING_GOES_HERE
  Mux : xbsIndex_r4/Mux  inputs=2  en=off  latency=0  precision=Full  arith_type=Unsigned  n_bits=16  bin_pt=14  quantization=Truncate  overflow=Wrap  dbl_ovrd=off  block_type=mux
  Relational : xbsIndex_r4/Relational  mode=a<=b  en=off  latency=0  dbl_ovrd=off  block_type=relational
      settings: xl_area=[0, 0, 0, 0, 0, 0, 0]  block_version=VER_STRING_GOES_HERE
  Relational1 : xbsIndex_r4/Relational  mode=a<b  en=off  latency=0  dbl_ovrd=off  block_type=relational
      settings: xl_area=[3 0 0 6 0 0 0]  block_version=VER_STRING_GOES_HERE
  Shift : xbsIndex_r4/Shift  shift_dir=Left  shift_bits=1  en=off  latency=0  precision=User Defined  arith_type=Unsigned  n_bits=1+ceil(log2(max_num_trainingSymbols))  bin_pt=0  quantization=Truncate  overflow=Flag as error  dbl_ovrd=off  block_type=shift
      settings: block_version=VER_STRING_GOES_HERE
  FirstSym : Outport
  Training : Outport  Port=2
  wires:
  [regRx_numTrainingSymbols] -> Shift/1, Mux/3
  SynIndex/1 -> Relational1/1, Relational/1
  Relational1/1 -> Training/1
  [regRx_AlamoutiMode] -> Mux/1
  Mux/1 -> Relational1/2
  Shift/1 -> Mux/2
  Logical2/1 -> Concat/1
  Clear2/1 -> Concat/2
  [regRx_SISOmode] -> Inverter1/1
  Concat/1 -> Relational/2
  Relational/1 -> FirstSym/1
  Inverter1/1 -> Logical2/2
  Inverter2/1 -> Logical2/1
  [regRx_AlamoutiMode] -> Inverter2/1

== system ofdm_txrx_supermimo/OFDM Rx MIMO/FFT & Chan Est/Channel Estimation & Pilot Processing/WE Gen ==
  note: SISOMode=1 writes estimates for antenna A for every
      | training symbol. SISOMode=0 (i.e. MIMO mode) alternates
      | between antenna A and B training symbols.
  note: "Swap" the AB and BA channel estimate
      | write enables in Alamouti mode.
      | Makes the math and control way
      | easier in the equalizer.
  Vin : Inport  OutDataType=sfix(16)  OutScaling=2^0
  Sym Index : Inport  Port=2  OutDataType=sfix(16)  OutScaling=2^0
  Train : Inport  Port=3  OutDataType=sfix(16)  OutScaling=2^0
  1LSB : xbsIndex_r4/Slice  nbits=1  boolean_output=on  mode=Lower Bit Location + Width  bit1=0  base1=MSB of Input  bit0=0  base0=LSB of Input  dbl_ovrd=off  block_type=slice
      settings: infoedit="Extracts a given range of bits from each input sample and presents it at the output.  The output type is ordinarily unsigned with binary point at zero, but can be Boolean when the slice is one bit wide.<P><P>Hardware notes: In hardware this block costs nothing."  block_version=VER_STRING_GOES_HERE
  1LSB+1 : xbsIndex_r4/Slice  nbits=1  boolean_output=on  mode=Lower Bit Location + Width  bit1=0  base1=MSB of Input  bit0=1  base0=LSB of Input  dbl_ovrd=off  block_type=slice
      settings: infoedit="Extracts a given range of bits from each input sample and presents it at the output.  The output type is ordinarily unsigned with binary point at zero, but can be Boolean when the slice is one bit wide.<P><P>Hardware notes: In hardware this block costs nothing."  block_version=VER_STRING_GOES_HERE
  Delay{1..4} (4 alike) : xbsIndex_r4/Delay  en=off  latency=1  dbl_ovrd=off  reg_retiming=on  block_type=delay
      settings: infoedit=Hardware notes: A delay line is a chain, each link of which is an SRL16 followed by a flip-flop. If register retiming is enabled, the delay line is a chain of flip-flops.  xl_area=[0, 0, 0, 0, 0, 0, 0]  block_version=VER_STRING_GOES_HERE
  Inverter{,1..4,7} (6 alike) : xbsIndex_r4/Inverter  en=off  latency=0  dbl_ovrd=off  block_type=inv
      Inverter settings: block_version=VER_STRING_GOES_HERE
      Inverter1 settings: block_version=VER_STRING_GOES_HERE
  Logical{,5..7} (4 alike) : xbsIndex_r4/Logical  logical_function=AND  inputs=4  en=off  latency=0  precision=Full  arith_type=Unsigned  n_bits=16  bin_pt=0  align_bp=on  dbl_ovrd=off  block_type=logical
  Logical1 : xbsIndex_r4/Logical  logical_function=AND  inputs=2  en=off  latency=0  precision=Full  arith_type=Unsigned  n_bits=8  bin_pt=2  align_bp=on  dbl_ovrd=off  block_type=logical
      settings: xl_area=[1 0 0 1 0 0 0]  block_version=VER_STRING_GOES_HERE
  Logical2 : xbsIndex_r4/Logical  logical_function=AND  inputs=2  en=off  latency=0  precision=Full  arith_type=Unsigned  n_bits=8  bin_pt=2  align_bp=on  dbl_ovrd=off  block_type=logical
      settings: xl_area=[1 0 0 1 0 0 0]  block_version=VER_STRING_GOES_HERE
  Logical3 : xbsIndex_r4/Logical  logical_function=OR  inputs=2  en=off  latency=0  precision=Full  arith_type=Unsigned  n_bits=8  bin_pt=2  align_bp=on  dbl_ovrd=off  block_type=logical
      settings: xl_area=[1 0 0 1 0 0 0]  block_version=VER_STRING_GOES_HERE
  Logical4 : xbsIndex_r4/Logical  logical_function=AND  inputs=2  en=off  latency=0  precision=Full  arith_type=Unsigned  n_bits=8  bin_pt=2  align_bp=on  dbl_ovrd=off  block_type=logical
      settings: xl_area=[1 0 0 1 0 0 0]  block_version=VER_STRING_GOES_HERE
  Seq0 : Outport  OutDataType=sfix(16)  OutScaling=2^0
  Seq1 : Outport  Port=2  OutDataType=sfix(16)  OutScaling=2^0
  Seq2 : Outport  Port=3  OutDataType=sfix(16)  OutScaling=2^0
  Seq3 : Outport  Port=4  OutDataType=sfix(16)  OutScaling=2^0
  wires:
  Inverter/1 -> Logical2/1
  1LSB+1/1 -> Logical2/2
  [regRx_SISOmode] -> Inverter/1
  Delay4/1 -> Seq3/1
  Delay2/1 -> Logical3/2
  Delay1/1 -> Logical1/2, Logical4/2
  Delay3/1 -> Seq0/1
  1LSB/1 -> Inverter7/1, Logical7/4, Inverter3/1, Logical5/4
  Vin/1 -> Logical6/1, Logical7/1, Logical5/1, Logical/1
  Logical7/1 -> Delay4/1
  Logical6/1 -> Delay2/1
  Logical5/1 -> Delay1/1
  Logical/1 -> Delay3/1
  Logical2/1 -> Logical6/3, Logical7/3, Inverter4/1, Inverter2/1
  Sym Index/1 -> 1LSB/1, 1LSB+1/1
  Train/1 -> Logical6/2, Logical7/2, Logical5/2, Logical/2
  [regRx_AlamoutiMode] -> Logical1/1, Inverter1/1
  Logical1/1 -> Logical3/1
  Logical3/1 -> Seq2/1
  Logical4/1 -> Seq1/1
  Inverter1/1 -> Logical4/1
  Inverter2/1 -> Logical/3
  Inverter3/1 -> Logical/4
  Inverter4/1 -> Logical5/3
  Inverter7/1 -> Logical6/4
